simulink library "Microgrid_Library"  (R2020a) (continued)
== system Microgrid_Library == (continued)
      mask DialogControl Container4 Prompt: House 1
      mask DialogControl Container10 Prompt: Kitchen
      mask DialogControl Container22 Prompt: Bathroom
      mask DialogControl Container20 Prompt: Bedroom 1
      mask DialogControl Container16 Prompt: Bedroom 2
      mask DialogControl Container11 Prompt: Lounge
      mask Display: image('house.jpg');
  "House 5 S  " : Subsystem  mask{V=240, t=60, K_L1_W=18, K_L1_On_1=23220, K_L1_Off_1=27120, K_L1_On_2=74160, K_L1_Off_2=82020, Dishwasher_W=1200, Dishwasher_On=36420, Fridge_W=150, T_Initial=1.35, T_Low=1.2, T_High=3.1, Jug_W=1500, Jug_On_1=25620, Jug_On_2=0, Jug_On_3=0, Toaster_W=1100, Toaster_On_1=30600, Toaster_On_2=0, N_Elements=4, Oven_On_1=65040, Oven_Off_1=69900, Shower_On_a=29100, Shower_L_a=240, Shower_On_b=39000, Shower_L_b=300, Shower_On_c=0, Shower_L_c=0, K_L3_W=18, K_L3_On_1=28800, K_L3_Off_1=29400, K_L3_On_2=38700, K_L3_Off_2=39120, HW_W=2000, M=135, D=0.488, L=1.35, Ti=7, Ta=20, T_Low_WH=56, T_High_WH=58, Clock_1_W=3, Lamp_1_W=18, L1_On_1=76560, L1_Off_1=82920, L1_On_2=0, L1_Off_2=0, K_L4_W=40, K_L4_On_1=73800, K_L4_Off_1=76200, Clock_2_W=5, Lamp_2_W=18, L2_On_1=26640, L2_Off_1=29520, L2_On_2=72000, L2_Off_2=79560, K_L5_W=60, K_L5_On_1=77100, K_L5_Off_1=79560, K_L5_On_2=0, K_L5_Off_2=0, Phone_C_1_W=5, Phone_C_1_On_a=0, Phone_C_1_Off_a=8760, Phone_C_1_On_b=77100, Phone_C_1_Off_b=86400, Phone_C_2_W=6, Phone_C_2_On_a=0, Phone_C_2_Off_a=21600, Phone_C_2_On_b=77100, Phone_C_2_Off_b=86400, Phone_C_3_W=5, Phone_C_3_On_a=0, Phone_C_3_Off_a=14820, Phone_C_3_On_b=77100, Phone_C_3_Off_b=86400, Laptop_C_1_W=65, Laptop_C_1_On_a=34200, Laptop_C_1_Off_a=52200, Laptop_C_2_W=65, Laptop_C_2_On_a=72780, Laptop_C_2_Off_a=77100, Laptop_C_2_On_b=0, Laptop_C_2_Off_b=0, Lamp_3_W=24, L3_On_1=25200, L3_Off_1=60450, L3_On_2=80040, L3_Off_2=84000, K_L6_W=100, K_L6_On_1=76200, K_L6_Off_1=69800, Heater_W=500, Heater_On_1=29160, Heater_Off_1=76140, Stereo_W=50, Stereo_On_1=43560, Stereo_Off_1=45480, TV_W=200, TV_On_1=39900, TV_Off_1=52200, TV_On_2=69600, TV_Off_2=77100}
      mask prompts: V "Voltage"; t "Time"; K_L1_W "Light_1_W"; K_L1_On_1 "Light_1_On_a"; K_L1_Off_1 "Light_1_Off_a"; K_L1_On_2 "Light_1_On_b"; K_L1_Off_2 "Light_1_Off_b"; T_Initial "Fridge_Initial_Temp"; T_Low "Fridge_T_Low"; T_High "Fridge_T_High"; N_Elements "Oven_#_Elements"; Shower_L_a "Shower_Length_a"; Shower_L_b "Shower_Length_b"; Shower_L_c "Shower_Length_c"; K_L3_W "Light_3_W"; K_L3_On_1 "Light_3_On_a"; K_L3_Off_1 "Light_3_Off_a"; K_L3_On_2 "Light_3_On_b"; K_L3_Off_2 "Light_3_Off_b"; M "Mass of water"; D "HW_Diameter"; L "HW_Length"; Ti "Inlet Temp"; Ta "Ambient Temp"; L1_On_1 "Lamp_1_On_1"; L1_Off_1 "Lamp_1_Off_1"; L1_On_2 "Lamp_1_On_2"; L1_Off_2 "Lamp_1_Off_2"; K_L4_W "Light_4_W"; K_L4_On_1 "Light_4_On_a"; K_L4_Off_1 "Light_4_Off_a"; L2_On_1 "Lamp_2_On_1"; L2_Off_1 "Lamp_2_Off_1"; L2_On_2 "Lamp_2_On_2"; L2_Off_2 "Lamp_2_Off_2"; K_L5_W "Light_5_W"; K_L5_On_1 "Light_5_On_a"; K_L5_Off_1 "Light_5_Off_a"; K_L5_On_2 "Light_5_On_b"; K_L5_Off_2 "Light_5_Off_b"; L3_On_1 "Lamp_3_On_1"; L3_Off_1 "Lamp_3_Off_1"; L3_On_2 "Lamp_3_On_2"; L3_Off_2 "Lamp_3_Off_2"; K_L6_W "Light_6_W"; K_L6_On_1 "Light_6_On_a"; K_L6_Off_1 "Light_6_Off_a"
      mask DialogControl Container4 Prompt: House 1
      mask DialogControl Container10 Prompt: Kitchen
      mask DialogControl Container22 Prompt: Bathroom
      mask DialogControl Container20 Prompt: Bedroom 1
      mask DialogControl Container16 Prompt: Bedroom 2
      mask DialogControl Container11 Prompt: Lounge
      mask Display: image('house.jpg');
  House 5 W : Subsystem  mask{V=240, t=60, K_L1_W=18, K_L1_On_1=23220, K_L1_Off_1=27120, K_L1_On_2=74160, K_L1_Off_2=82020, Dishwasher_W=1200, Dishwasher_On=36420, Fridge_W=150, T_Initial=1.35, T_Low=1.2, T_High=3.1, Jug_W=1500, Jug_On_1=25620, Jug_On_2=0, Jug_On_3=0, Toaster_W=1100, Toaster_On_1=30600, Toaster_On_2=0, N_Elements=4, Oven_On_1=65040, Oven_Off_1=69900, Shower_On_a=29100, Shower_L_a=240, Shower_On_b=39000, Shower_L_b=300, Shower_On_c=0, Shower_L_c=0, K_L3_W=18, K_L3_On_1=28800, K_L3_Off_1=29400, K_L3_On_2=38700, K_L3_Off_2=39120, HW_W=2000, M=135, D=0.488, L=1.35, Ti=7, Ta=20, T_Low_WH=56, T_High_WH=58, Clock_1_W=3, Lamp_1_W=18, L1_On_1=70560, L1_Off_1=82920, L1_On_2=0, L1_Off_2=0, K_L4_W=40, K_L4_On_1=65400, K_L4_Off_1=76200, Clock_2_W=5, Lamp_2_W=18, L2_On_1=26640, L2_Off_1=29520, L2_On_2=66000, L2_Off_2=79560, K_L5_W=60, K_L5_On_1=77100, K_L5_Off_1=79560, K_L5_On_2=0, K_L5_Off_2=0, Phone_C_1_W=5, Phone_C_1_On_a=0, Phone_C_1_Off_a=8760, Phone_C_1_On_b=77100, Phone_C_1_Off_b=86400, Phone_C_2_W=6, Phone_C_2_On_a=0, Phone_C_2_Off_a=21600, Phone_C_2_On_b=77100, Phone_C_2_Off_b=86400, Phone_C_3_W=5, Phone_C_3_On_a=0, Phone_C_3_Off_a=14820, Phone_C_3_On_b=77100, Phone_C_3_Off_b=86400, Laptop_C_1_W=65, Laptop_C_1_On_a=34200, Laptop_C_1_Off_a=52200, Laptop_C_2_W=65, Laptop_C_2_On_a=72780, Laptop_C_2_Off_a=77100, Laptop_C_2_On_b=0, Laptop_C_2_Off_b=0, Lamp_3_W=24, L3_On_1=25200, L3_Off_1=27000, L3_On_2=20040, L3_Off_2=84000, K_L6_W=100, K_L6_On_1=70200, K_L6_Off_1=73800, Heater_W=2000, Heater_On_1=29160, Heater_Off_1=76140, Stereo_W=50, Stereo_On_1=43560, Stereo_Off_1=45480, TV_W=200, TV_On_1=39900, TV_Off_1=52200, TV_On_2=69600, TV_Off_2=77100}
      mask prompts: V "Voltage"; t "Time"; K_L1_W "Light_1_W"; K_L1_On_1 "Light_1_On_a"; K_L1_Off_1 "Light_1_Off_a"; K_L1_On_2 "Light_1_On_b"; K_L1_Off_2 "Light_1_Off_b"; T_Initial "Fridge_Initial_Temp"; T_Low "Fridge_T_Low"; T_High "Fridge_T_High"; N_Elements "Oven_#_Elements"; Shower_L_a "Shower_Length_a"; Shower_L_b "Shower_Length_b"; Shower_L_c "Shower_Length_c"; K_L3_W "Light_3_W"; K_L3_On_1 "Light_3_On_a"; K_L3_Off_1 "Light_3_Off_a"; K_L3_On_2 "Light_3_On_b"; K_L3_Off_2 "Light_3_Off_b"; M "Mass of water"; D "HW_Diameter"; L "HW_Length"; Ti "Inlet Temp"; Ta "Ambient Temp"; L1_On_1 "Lamp_1_On_1"; L1_Off_1 "Lamp_1_Off_1"; L1_On_2 "Lamp_1_On_2"; L1_Off_2 "Lamp_1_Off_2"; K_L4_W "Light_4_W"; K_L4_On_1 "Light_4_On_a"; K_L4_Off_1 "Light_4_Off_a"; L2_On_1 "Lamp_2_On_1"; L2_Off_1 "Lamp_2_Off_1"; L2_On_2 "Lamp_2_On_2"; L2_Off_2 "Lamp_2_Off_2"; K_L5_W "Light_5_W"; K_L5_On_1 "Light_5_On_a"; K_L5_Off_1 "Light_5_Off_a"; K_L5_On_2 "Light_5_On_b"; K_L5_Off_2 "Light_5_Off_b"; L3_On_1 "Lamp_3_On_1"; L3_Off_1 "Lamp_3_Off_1"; L3_On_2 "Lamp_3_On_2"; L3_Off_2 "Lamp_3_Off_2"; K_L6_W "Light_6_W"; K_L6_On_1 "Light_6_On_a"; K_L6_Off_1 "Light_6_Off_a"
      mask DialogControl Container4 Prompt: House 1
      mask DialogControl Container10 Prompt: Kitchen
      mask DialogControl Container22 Prompt: Bathroom
      mask DialogControl Container20 Prompt: Bedroom 1
      mask DialogControl Container16 Prompt: Bedroom 2
      mask DialogControl Container11 Prompt: Lounge
      mask Display: image('house.jpg');
  House 6 S : Subsystem  mask{V=240, t=60, K_L1_W=40, K_L1_On_1=75000, K_L1_Off_1=81300, K_L1_On_2=0, K_L1_Off_2=0, Dishwasher_W=1200, Dishwasher_On=69000, Fridge_W=360, T_Initial=1.8, T_Low=1.5, T_High=2.4, Jug_W=2000, Jug_On_1=30540, Jug_On_2=70680, Jug_On_3=0, Toaster_W=1200, Toaster_On_1=28800, Toaster_On_2=29100, N_Elements=4, Oven_On_1=61260, Oven_Off_1=65160, Shower_On_a=68760, Shower_L_a=300, Shower_On_b=77520, Shower_L_b=180, Shower_On_c=78780, Shower_L_c=300, K_L3_W=24, K_L3_On_1=68700, K_L3_Off_1=72000, K_L3_On_2=77400, K_L3_Off_2=79200, HW_W=3000, M=300, D=0.58, L=1.825, Ti=7, Ta=20, T_Low_WH=55, T_High_WH=60, Clock_1_W=6, Lamp_1_W=24, L1_On_1=21600, L1_Off_1=27000, L1_On_2=0, L1_Off_2=0, K_L4_W=0, K_L4_On_1=0, K_L4_Off_1=0, Clock_2_W=5, Lamp_2_W=24, L2_On_1=21600, L2_Off_1=27000, L2_On_2=74280, L2_Off_2=81300, K_L5_W=40, K_L5_On_1=0, K_L5_Off_1=3240, K_L5_On_2=78600, K_L5_Off_2=86400, Phone_C_1_W=6, Phone_C_1_On_a=57600, Phone_C_1_Off_a=81600, Phone_C_1_On_b=0, Phone_C_1_Off_b=0, Phone_C_2_W=5, Phone_C_2_On_a=0, Phone_C_2_Off_a=12000, Phone_C_2_On_b=84000, Phone_C_2_Off_b=86400, Phone_C_3_W=0, Phone_C_3_On_a=0, Phone_C_3_Off_a=0, Phone_C_3_On_b=0, Phone_C_3_Off_b=0, Laptop_C_1_W=65, Laptop_C_1_On_a=75600, Laptop_C_1_Off_a=79560, Laptop_C_2_W=55, Laptop_C_2_On_a=0, Laptop_C_2_Off_a=3120, Laptop_C_2_On_b=79440, Laptop_C_2_Off_b=86400, Lamp_3_W=40, L3_On_1=21720, L3_Off_1=30000, L3_On_2=72000, L3_Off_2=81000, K_L6_W=60, K_L6_On_1=72000, K_L6_Off_1=81000, Heater_W=2100, Heater_On_1=56340, Heater_Off_1=73020, Stereo_W=100, Stereo_On_1=63000, Stereo_Off_1=64800, TV_W=400, TV_On_1=22020, TV_Off_1=70200, TV_On_2=70200, TV_Off_2=78600}
      mask prompts: V "Voltage"; t "Time"; K_L1_W "Light_1_W"; K_L1_On_1 "Light_1_On_a"; K_L1_Off_1 "Light_1_Off_a"; K_L1_On_2 "Light_1_On_b"; K_L1_Off_2 "Light_1_Off_b"; T_Initial "Fridge_Initial_Temp"; T_Low "Fridge_T_Low"; T_High "Fridge_T_High"; N_Elements "Oven_#_Elements"; Shower_L_a "Shower_Length_a"; Shower_L_b "Shower_Length_b"; Shower_L_c "Shower_Length_c"; K_L3_W "Light_3_W"; K_L3_On_1 "Light_3_On_a"; K_L3_Off_1 "Light_3_Off_a"; K_L3_On_2 "Light_3_On_b"; K_L3_Off_2 "Light_3_Off_b"; M "Mass of water"; D "HW_Diameter"; L "HW_Length"; Ti "Inlet Temp"; Ta "Ambient Temp"; L1_On_1 "Lamp_1_On_1"; L1_Off_1 "Lamp_1_Off_1"; L1_On_2 "Lamp_1_On_2"; L1_Off_2 "Lamp_1_Off_2"; K_L4_W "Light_4_W"; K_L4_On_1 "Light_4_On_a"; K_L4_Off_1 "Light_4_Off_a"; L2_On_1 "Lamp_2_On_1"; L2_Off_1 "Lamp_2_Off_1"; L2_On_2 "Lamp_2_On_2"; L2_Off_2 "Lamp_2_Off_2"; K_L5_W "Light_5_W"; K_L5_On_1 "Light_5_On_a"; K_L5_Off_1 "Light_5_Off_a"; K_L5_On_2 "Light_5_On_b"; K_L5_Off_2 "Light_5_Off_b"; L3_On_1 "Lamp_3_On_1"; L3_Off_1 "Lamp_3_Off_1"; L3_On_2 "Lamp_3_On_2"; L3_Off_2 "Lamp_3_Off_2"; K_L6_W "Light_6_W"; K_L6_On_1 "Light_6_On_a"; K_L6_Off_1 "Light_6_Off_a"
      mask DialogControl Container4 Prompt: House 1
      mask DialogControl Container10 Prompt: Kitchen
      mask DialogControl Container22 Prompt: Bathroom
      mask DialogControl Container20 Prompt: Bedroom 1
      mask DialogControl Container16 Prompt: Bedroom 2
      mask DialogControl Container11 Prompt: Lounge
      mask Display: image('house.jpg');
  House 6 W : Subsystem  mask{V=240, t=60, K_L1_W=40, K_L1_On_1=63000, K_L1_Off_1=69300, K_L1_On_2=0, K_L1_Off_2=0, Dishwasher_W=1200, Dishwasher_On=69000, Fridge_W=360, T_Initial=1.8, T_Low=1.5, T_High=2.4, Jug_W=2000, Jug_On_1=30540, Jug_On_2=70680, Jug_On_3=0, Toaster_W=1200, Toaster_On_1=28800, Toaster_On_2=29100, N_Elements=4, Oven_On_1=61260, Oven_Off_1=65160, Shower_On_a=68760, Shower_L_a=300, Shower_On_b=77520, Shower_L_b=180, Shower_On_c=78780, Shower_L_c=300, K_L3_W=24, K_L3_On_1=68700, K_L3_Off_1=72000, K_L3_On_2=77400, K_L3_Off_2=79200, HW_W=3000, M=300, D=0.58, L=1.825, Ti=7, Ta=20, T_Low_WH=55, T_High_WH=60, Clock_1_W=6, Lamp_1_W=24, L1_On_1=21600, L1_Off_1=27000, L1_On_2=0, L1_Off_2=0, K_L4_W=40, K_L4_On_1=1150, K_L4_Off_1=1200, Clock_2_W=5, Lamp_2_W=24, L2_On_1=21600, L2_Off_1=27000, L2_On_2=74280, L2_Off_2=81300, K_L5_W=40, K_L5_On_1=0, K_L5_Off_1=3240, K_L5_On_2=78600, K_L5_Off_2=86400, Phone_C_1_W=6, Phone_C_1_On_a=57600, Phone_C_1_Off_a=81600, Phone_C_1_On_b=0, Phone_C_1_Off_b=0, Phone_C_2_W=5, Phone_C_2_On_a=0, Phone_C_2_Off_a=12000, Phone_C_2_On_b=84000, Phone_C_2_Off_b=86400, Phone_C_3_W=0, Phone_C_3_On_a=0, Phone_C_3_Off_a=0, Phone_C_3_On_b=0, Phone_C_3_Off_b=0, Laptop_C_1_W=65, Laptop_C_1_On_a=75600, Laptop_C_1_Off_a=79560, Laptop_C_2_W=55, Laptop_C_2_On_a=0, Laptop_C_2_Off_a=3120, Laptop_C_2_On_b=79440, Laptop_C_2_Off_b=86400, Lamp_3_W=40, L3_On_1=21720, L3_Off_1=30000, L3_On_2=66000, L3_Off_2=81000, K_L6_W=60, K_L6_On_1=66000, K_L6_Off_1=81000, Heater_W=2100, Heater_On_1=56340, Heater_Off_1=73020, Stereo_W=100, Stereo_On_1=63000, Stereo_Off_1=64800, TV_W=400, TV_On_1=22020, TV_Off_1=70200, TV_On_2=70200, TV_Off_2=78600}
      mask prompts: V "Voltage"; t "Time"; K_L1_W "Light_1_W"; K_L1_On_1 "Light_1_On_a"; K_L1_Off_1 "Light_1_Off_a"; K_L1_On_2 "Light_1_On_b"; K_L1_Off_2 "Light_1_Off_b"; T_Initial "Fridge_Initial_Temp"; T_Low "Fridge_T_Low"; T_High "Fridge_T_High"; N_Elements "Oven_#_Elements"; Shower_L_a "Shower_Length_a"; Shower_L_b "Shower_Length_b"; Shower_L_c "Shower_Length_c"; K_L3_W "Light_3_W"; K_L3_On_1 "Light_3_On_a"; K_L3_Off_1 "Light_3_Off_a"; K_L3_On_2 "Light_3_On_b"; K_L3_Off_2 "Light_3_Off_b"; M "Mass of water"; D "HW_Diameter"; L "HW_Length"; Ti "Inlet Temp"; Ta "Ambient Temp"; L1_On_1 "Lamp_1_On_1"; L1_Off_1 "Lamp_1_Off_1"; L1_On_2 "Lamp_1_On_2"; L1_Off_2 "Lamp_1_Off_2"; K_L4_W "Light_4_W"; K_L4_On_1 "Light_4_On_a"; K_L4_Off_1 "Light_4_Off_a"; L2_On_1 "Lamp_2_On_1"; L2_Off_1 "Lamp_2_Off_1"; L2_On_2 "Lamp_2_On_2"; L2_Off_2 "Lamp_2_Off_2"; K_L5_W "Light_5_W"; K_L5_On_1 "Light_5_On_a"; K_L5_Off_1 "Light_5_Off_a"; K_L5_On_2 "Light_5_On_b"; K_L5_Off_2 "Light_5_Off_b"; L3_On_1 "Lamp_3_On_1"; L3_Off_1 "Lamp_3_Off_1"; L3_On_2 "Lamp_3_On_2"; L3_Off_2 "Lamp_3_Off_2"; K_L6_W "Light_6_W"; K_L6_On_1 "Light_6_On_a"; K_L6_Off_1 "Light_6_Off_a"
      mask DialogControl Container4 Prompt: House 1
      mask DialogControl Container10 Prompt: Kitchen
      mask DialogControl Container22 Prompt: Bathroom
      mask DialogControl Container20 Prompt: Bedroom 1
      mask DialogControl Container16 Prompt: Bedroom 2
      mask DialogControl Container11 Prompt: Lounge
      mask Display: image('house.jpg');
  House 7 S : Subsystem  mask{V=240, t=60, K_L1_W=60, K_L1_On_1=27120, K_L1_Off_1=27780, K_L1_On_2=73140, K_L1_Off_2=78000, Dishwasher_W=1200, Dishwasher_On=28800, Fridge_W=200, T_Initial=1, T_Low=1.3, T_High=2, Jug_W=1500, Jug_On_1=28980, Jug_On_2=48060, Jug_On_3=0, Toaster_W=1100, Toaster_On_1=64800, Toaster_On_2=0, N_Elements=3, Oven_On_1=68640, Oven_Off_1=70860, Shower_On_a=30720, Shower_L_a=300, Shower_On_b=75450, Shower_L_b=240, Shower_On_c=0, Shower_L_c=0, K_L3_W=40, K_L3_On_1=72120, K_L3_Off_1=72600, K_L3_On_2=0, K_L3_Off_2=0, HW_W=2000, M=180, D=0.58, L=1.166, Ti=7, Ta=20, T_Low_WH=57, T_High_WH=62, Clock_1_W=4, Lamp_1_W=24, L1_On_1=72540, L1_Off_1=72900, L1_On_2=72540, L1_Off_2=72900, K_L4_W=0, K_L4_On_1=0, K_L4_Off_1=0, Clock_2_W=4, Lamp_2_W=40, L2_On_1=27000, L2_Off_1=31560, L2_On_2=77160, L2_Off_2=85200, K_L5_W=80, K_L5_On_1=27000, K_L5_Off_1=28200, K_L5_On_2=83160, K_L5_Off_2=83940, Phone_C_1_W=4, Phone_C_1_On_a=0, Phone_C_1_Off_a=28380, Phone_C_1_On_b=78900, Phone_C_1_Off_b=86400, Phone_C_2_W=6, Phone_C_2_On_a=30240, Phone_C_2_Off_a=51840, Phone_C_2_On_b=0, Phone_C_2_Off_b=0, Phone_C_3_W=4, Phone_C_3_On_a=0, Phone_C_3_Off_a=24360, Phone_C_3_On_b=85200, Phone_C_3_Off_b=86400, Laptop_C_1_W=55, Laptop_C_1_On_a=36240, Laptop_C_1_Off_a=50400, Laptop_C_2_W=45, Laptop_C_2_On_a=0, Laptop_C_2_Off_a=22860, Laptop_C_2_On_b=84600, Laptop_C_2_Off_b=86400, Lamp_3_W=0, L3_On_1=0, L3_Off_1=0, L3_On_2=0, L3_Off_2=0, K_L6_W=18, K_L6_On_1=71940, K_L6_Off_1=79200, Heater_W=1000, Heater_On_1=54000, Heater_Off_1=73800, Stereo_W=50, Stereo_On_1=36540, Stereo_Off_1=50100, TV_W=100, TV_On_1=55260, TV_Off_1=57780, TV_On_2=72000, TV_Off_2=75840}
      mask prompts: V "Voltage"; t "Time"; K_L1_W "Light_1_W"; K_L1_On_1 "Light_1_On_a"; K_L1_Off_1 "Light_1_Off_a"; K_L1_On_2 "Light_1_On_b"; K_L1_Off_2 "Light_1_Off_b"; T_Initial "Fridge_Initial_Temp"; T_Low "Fridge_T_Low"; T_High "Fridge_T_High"; N_Elements "Oven_#_Elements"; Shower_L_a "Shower_Length_a"; Shower_L_b "Shower_Length_b"; Shower_L_c "Shower_Length_c"; K_L3_W "Light_3_W"; K_L3_On_1 "Light_3_On_a"; K_L3_Off_1 "Light_3_Off_a"; K_L3_On_2 "Light_3_On_b"; K_L3_Off_2 "Light_3_Off_b"; M "Mass of water"; D "HW_Diameter"; L "HW_Length"; Ti "Inlet Temp"; Ta "Ambient Temp"; L1_On_1 "Lamp_1_On_1"; L1_Off_1 "Lamp_1_Off_1"; L1_On_2 "Lamp_1_On_2"; L1_Off_2 "Lamp_1_Off_2"; K_L4_W "Light_4_W"; K_L4_On_1 "Light_4_On_a"; K_L4_Off_1 "Light_4_Off_a"; L2_On_1 "Lamp_2_On_1"; L2_Off_1 "Lamp_2_Off_1"; L2_On_2 "Lamp_2_On_2"; L2_Off_2 "Lamp_2_Off_2"; K_L5_W "Light_5_W"; K_L5_On_1 "Light_5_On_a"; K_L5_Off_1 "Light_5_Off_a"; K_L5_On_2 "Light_5_On_b"; K_L5_Off_2 "Light_5_Off_b"; L3_On_1 "Lamp_3_On_1"; L3_Off_1 "Lamp_3_Off_1"; L3_On_2 "Lamp_3_On_2"; L3_Off_2 "Lamp_3_Off_2"; K_L6_W "Light_6_W"; K_L6_On_1 "Light_6_On_a"; K_L6_Off_1 "Light_6_Off_a"
      mask DialogControl Container4 Prompt: House 1
      mask DialogControl Container10 Prompt: Kitchen
      mask DialogControl Container22 Prompt: Bathroom
      mask DialogControl Container20 Prompt: Bedroom 1
      mask DialogControl Container16 Prompt: Bedroom 2
      mask DialogControl Container11 Prompt: Lounge
      mask Display: image('house.jpg');
  House 7 W : Subsystem  mask{V=240, t=60, K_L1_W=60, K_L1_On_1=27120, K_L1_Off_1=27780, K_L1_On_2=67140, K_L1_Off_2=72000, Dishwasher_W=1200, Dishwasher_On=28800, Fridge_W=200, T_Initial=1, T_Low=1.3, T_High=2, Jug_W=1500, Jug_On_1=28980, Jug_On_2=48060, Jug_On_3=0, Toaster_W=1100, Toaster_On_1=64800, Toaster_On_2=0, N_Elements=3, Oven_On_1=68640, Oven_Off_1=70860, Shower_On_a=30720, Shower_L_a=300, Shower_On_b=75450, Shower_L_b=240, Shower_On_c=0, Shower_L_c=0, K_L3_W=40, K_L3_On_1=72120, K_L3_Off_1=72600, K_L3_On_2=0, K_L3_Off_2=0, HW_W=2000, M=180, D=0.58, L=1.166, Ti=7, Ta=20, T_Low_WH=57, T_High_WH=62, Clock_1_W=4, Lamp_1_W=24, L1_On_1=72540, L1_Off_1=72900, L1_On_2=72540, L1_Off_2=72900, K_L4_W=0, K_L4_On_1=0, K_L4_Off_1=0, Clock_2_W=4, Lamp_2_W=40, L2_On_1=27000, L2_Off_1=31560, L2_On_2=77160, L2_Off_2=85200, K_L5_W=80, K_L5_On_1=27000, K_L5_Off_1=28200, K_L5_On_2=83160, K_L5_Off_2=83940, Phone_C_1_W=4, Phone_C_1_On_a=0, Phone_C_1_Off_a=28380, Phone_C_1_On_b=78900, Phone_C_1_Off_b=86400, Phone_C_2_W=6, Phone_C_2_On_a=30240, Phone_C_2_Off_a=51840, Phone_C_2_On_b=0, Phone_C_2_Off_b=0, Phone_C_3_W=4, Phone_C_3_On_a=0, Phone_C_3_Off_a=24360, Phone_C_3_On_b=85200, Phone_C_3_Off_b=86400, Laptop_C_1_W=55, Laptop_C_1_On_a=36240, Laptop_C_1_Off_a=50400, Laptop_C_2_W=45, Laptop_C_2_On_a=0, Laptop_C_2_Off_a=22860, Laptop_C_2_On_b=84600, Laptop_C_2_Off_b=86400, Lamp_3_W=0, L3_On_1=0, L3_Off_1=0, L3_On_2=0, L3_Off_2=0, K_L6_W=18, K_L6_On_1=71940, K_L6_Off_1=79200, Heater_W=1000, Heater_On_1=54000, Heater_Off_1=73800, Stereo_W=50, Stereo_On_1=36540, Stereo_Off_1=50100, TV_W=100, TV_On_1=55260, TV_Off_1=57780, TV_On_2=72000, TV_Off_2=75840}
      mask prompts: V "Voltage"; t "Time"; K_L1_W "Light_1_W"; K_L1_On_1 "Light_1_On_a"; K_L1_Off_1 "Light_1_Off_a"; K_L1_On_2 "Light_1_On_b"; K_L1_Off_2 "Light_1_Off_b"; T_Initial "Fridge_Initial_Temp"; T_Low "Fridge_T_Low"; T_High "Fridge_T_High"; N_Elements "Oven_#_Elements"; Shower_L_a "Shower_Length_a"; Shower_L_b "Shower_Length_b"; Shower_L_c "Shower_Length_c"; K_L3_W "Light_3_W"; K_L3_On_1 "Light_3_On_a"; K_L3_Off_1 "Light_3_Off_a"; K_L3_On_2 "Light_3_On_b"; K_L3_Off_2 "Light_3_Off_b"; M "Mass of water"; D "HW_Diameter"; L "HW_Length"; Ti "Inlet Temp"; Ta "Ambient Temp"; L1_On_1 "Lamp_1_On_1"; L1_Off_1 "Lamp_1_Off_1"; L1_On_2 "Lamp_1_On_2"; L1_Off_2 "Lamp_1_Off_2"; K_L4_W "Light_4_W"; K_L4_On_1 "Light_4_On_a"; K_L4_Off_1 "Light_4_Off_a"; L2_On_1 "Lamp_2_On_1"; L2_Off_1 "Lamp_2_Off_1"; L2_On_2 "Lamp_2_On_2"; L2_Off_2 "Lamp_2_Off_2"; K_L5_W "Light_5_W"; K_L5_On_1 "Light_5_On_a"; K_L5_Off_1 "Light_5_Off_a"; K_L5_On_2 "Light_5_On_b"; K_L5_Off_2 "Light_5_Off_b"; L3_On_1 "Lamp_3_On_1"; L3_Off_1 "Lamp_3_Off_1"; L3_On_2 "Lamp_3_On_2"; L3_Off_2 "Lamp_3_Off_2"; K_L6_W "Light_6_W"; K_L6_On_1 "Light_6_On_a"; K_L6_Off_1 "Light_6_Off_a"
      mask DialogControl Container4 Prompt: House 1
      mask DialogControl Container10 Prompt: Kitchen
      mask DialogControl Container22 Prompt: Bathroom
      mask DialogControl Container20 Prompt: Bedroom 1
      mask DialogControl Container16 Prompt: Bedroom 2
      mask DialogControl Container11 Prompt: Lounge
      mask Display: image('house.jpg');
  House 8 S : Subsystem  mask{V=240, t=60, K_L1_W=24, K_L1_On_1=75480, K_L1_Off_1=81540, K_L1_On_2=0, K_L1_Off_2=0, K_L2_W=60, K_L2_On_1=27540, K_L2_Off_1=31440, Fridge_W=200, T_Initial=1.7, T_Low=0.9, T_High=3.6, Jug_W=2000, Jug_On_1=32580, Jug_On_2=0, Jug_On_3=0, Toaster_W=1100, Toaster_On_1=33120, Toaster_On_2=0, N_Elements=4, Oven_On_1=64420, Oven_Off_1=67440, Shower_On_a=31200, Shower_L_a=240, Shower_On_b=68700, Shower_L_b=300, Shower_On_c=83340, Shower_L_c=180, K_L3_W=100, K_L3_On_1=68400, K_L3_Off_1=69000, K_L3_On_2=83280, K_L3_Off_2=83700, HW_W=3000, M=250, D=0.58, L=1.56, Ti=7, Ta=20, T_Low_WH=55.1, T_High_WH=64, Clock_1_W=5, Lamp_1_W=24, L1_On_1=74400, L1_Off_1=75000, L1_On_2=0, L1_Off_2=0, K_L4_W=40, K_L4_On_1=21900, K_L4_Off_1=27240, Clock_2_W=6, Lamp_2_W=60, L2_On_1=0, L2_Off_1=1380, L2_On_2=84180, L2_Off_2=86400, K_L5_W=24, K_L5_On_1=22200, K_L5_Off_1=24300, K_L5_On_2=83160, K_L5_Off_2=84180, Phone_C_1_W=7, Phone_C_1_On_a=0, Phone_C_1_Off_a=31920, Phone_C_1_On_b=76440, Phone_C_1_Off_b=86400, Phone_C_2_W=4, Phone_C_2_On_a=0, Phone_C_2_Off_a=18600, Phone_C_2_On_b=79140, Phone_C_2_Off_b=86400, Phone_C_3_W=0, Phone_C_3_On_a=0, Phone_C_3_Off_a=0, Phone_C_3_On_b=0, Phone_C_3_Off_b=0, Laptop_C_1_W=65, Laptop_C_1_On_a=22800, Laptop_C_1_Off_a=85020, Laptop_C_2_W=45, Laptop_C_2_On_a=0, Laptop_C_2_Off_a=27360, Laptop_C_2_On_b=78720, Laptop_C_2_Off_b=86400, Lamp_3_W=40, L3_On_1=22080, L3_Off_1=27000, L3_On_2=72480, L3_Off_2=78360, K_L6_W=24, K_L6_On_1=78360, K_L6_Off_1=8400, Heater_W=2000, Heater_On_1=58320, Heater_Off_1=72360, Stereo_W=80, Stereo_On_1=57600, Stereo_Off_1=64800, TV_W=200, TV_On_1=23820, TV_Off_1=27000, TV_On_2=73980, TV_Off_2=83160}
      mask prompts: V "Voltage"; t "Time"; K_L1_W "Light_1_W"; K_L1_On_1 "Light_1_On_a"; K_L1_Off_1 "Light_1_Off_a"; K_L1_On_2 "Light_1_On_b"; K_L1_Off_2 "Light_1_Off_b"; K_L2_W "Light_2_W"; K_L2_On_1 "Light_2_On_a"; K_L2_Off_1 "Light_2_Off_a"; T_Initial "Fridge_Initial_Temp"; T_Low "Fridge_T_Low"; T_High "Fridge_T_High"; N_Elements "Oven_#_Elements"; Shower_L_a "Shower_Length_a"; Shower_L_b "Shower_Length_b"; Shower_L_c "Shower_Length_c"; K_L3_W "Light_3_W"; K_L3_On_1 "Light_3_On_a"; K_L3_Off_1 "Light_3_Off_a"; K_L3_On_2 "Light_3_On_b"; K_L3_Off_2 "Light_3_Off_b"; M "Mass of water"; D "HW_Diameter"; L "HW_Length"; Ti "Inlet Temp"; Ta "Ambient Temp"; L1_On_1 "Lamp_1_On_1"; L1_Off_1 "Lamp_1_Off_1"; L1_On_2 "Lamp_1_On_2"; L1_Off_2 "Lamp_1_Off_2"; K_L4_W "Light_4_W"; K_L4_On_1 "Light_4_On_a"; K_L4_Off_1 "Light_4_Off_a"; L2_On_1 "Lamp_2_On_1"; L2_Off_1 "Lamp_2_Off_1"; L2_On_2 "Lamp_2_On_2"; L2_Off_2 "Lamp_2_Off_2"; K_L5_W "Light_5_W"; K_L5_On_1 "Light_5_On_a"; K_L5_Off_1 "Light_5_Off_a"; K_L5_On_2 "Light_5_On_b"; K_L5_Off_2 "Light_5_Off_b"; L3_On_1 "Lamp_3_On_1"; L3_Off_1 "Lamp_3_Off_1"; L3_On_2 "Lamp_3_On_2"; L3_Off_2 "Lamp_3_Off_2"; K_L6_W "Light_6_W"; K_L6_On_1 "Light_6_On_a"; K_L6_Off_1 "Light_6_Off_a"
      mask DialogControl Container4 Prompt: House 1
      mask DialogControl Container10 Prompt: Kitchen
      mask DialogControl Container22 Prompt: Bathroom
      mask DialogControl Container20 Prompt: Bedroom 1
      mask DialogControl Container16 Prompt: Bedroom 2
      mask DialogControl Container11 Prompt: Lounge
      mask Display: image('house.jpg');
  House 8 W : Subsystem  mask{V=240, t=60, K_L1_W=24, K_L1_On_1=63480, K_L1_Off_1=69540, K_L1_On_2=0, K_L1_Off_2=0, K_L2_W=60, K_L2_On_1=27540, K_L2_Off_1=31440, Fridge_W=200, T_Initial=1.7, T_Low=0.9, T_High=3.6, Jug_W=2000, Jug_On_1=32580, Jug_On_2=0, Jug_On_3=0, Toaster_W=1100, Toaster_On_1=33120, Toaster_On_2=0, N_Elements=4, Oven_On_1=64420, Oven_Off_1=67440, Shower_On_a=31200, Shower_L_a=240, Shower_On_b=68700, Shower_L_b=300, Shower_On_c=83340, Shower_L_c=180, K_L3_W=100, K_L3_On_1=68400, K_L3_Off_1=69000, K_L3_On_2=83280, K_L3_Off_2=83700, HW_W=3000, M=250, D=0.58, L=1.56, Ti=7, Ta=20, T_Low_WH=55.1, T_High_WH=64, Clock_1_W=5, Lamp_1_W=24, L1_On_1=68400, L1_Off_1=69000, L1_On_2=0, L1_Off_2=0, K_L4_W=40, K_L4_On_1=21900, K_L4_Off_1=27240, Clock_2_W=6, Lamp_2_W=60, L2_On_1=0, L2_Off_1=1380, L2_On_2=84180, L2_Off_2=86400, K_L5_W=24, K_L5_On_1=22200, K_L5_Off_1=24300, K_L5_On_2=83160, K_L5_Off_2=84180, Phone_C_1_W=7, Phone_C_1_On_a=0, Phone_C_1_Off_a=31920, Phone_C_1_On_b=76440, Phone_C_1_Off_b=86400, Phone_C_2_W=4, Phone_C_2_On_a=0, Phone_C_2_Off_a=18600, Phone_C_2_On_b=79140, Phone_C_2_Off_b=86400, Phone_C_3_W=0, Phone_C_3_On_a=0, Phone_C_3_Off_a=0, Phone_C_3_On_b=0, Phone_C_3_Off_b=0, Laptop_C_1_W=65, Laptop_C_1_On_a=22800, Laptop_C_1_Off_a=85020, Laptop_C_2_W=45, Laptop_C_2_On_a=0, Laptop_C_2_Off_a=27360, Laptop_C_2_On_b=78720, Laptop_C_2_Off_b=86400, Lamp_3_W=40, L3_On_1=22080, L3_Off_1=27000, L3_On_2=72480, L3_Off_2=78360, K_L6_W=24, K_L6_On_1=78360, K_L6_Off_1=8400, Heater_W=2000, Heater_On_1=58320, Heater_Off_1=72360, Stereo_W=80, Stereo_On_1=57600, Stereo_Off_1=64800, TV_W=200, TV_On_1=23820, TV_Off_1=27000, TV_On_2=73980, TV_Off_2=83160}
      mask prompts: V "Voltage"; t "Time"; K_L1_W "Light_1_W"; K_L1_On_1 "Light_1_On_a"; K_L1_Off_1 "Light_1_Off_a"; K_L1_On_2 "Light_1_On_b"; K_L1_Off_2 "Light_1_Off_b"; K_L2_W "Light_2_W"; K_L2_On_1 "Light_2_On_a"; K_L2_Off_1 "Light_2_Off_a"; T_Initial "Fridge_Initial_Temp"; T_Low "Fridge_T_Low"; T_High "Fridge_T_High"; N_Elements "Oven_#_Elements"; Shower_L_a "Shower_Length_a"; Shower_L_b "Shower_Length_b"; Shower_L_c "Shower_Length_c"; K_L3_W "Light_3_W"; K_L3_On_1 "Light_3_On_a"; K_L3_Off_1 "Light_3_Off_a"; K_L3_On_2 "Light_3_On_b"; K_L3_Off_2 "Light_3_Off_b"; M "Mass of water"; D "HW_Diameter"; L "HW_Length"; Ti "Inlet Temp"; Ta "Ambient Temp"; L1_On_1 "Lamp_1_On_1"; L1_Off_1 "Lamp_1_Off_1"; L1_On_2 "Lamp_1_On_2"; L1_Off_2 "Lamp_1_Off_2"; K_L4_W "Light_4_W"; K_L4_On_1 "Light_4_On_a"; K_L4_Off_1 "Light_4_Off_a"; L2_On_1 "Lamp_2_On_1"; L2_Off_1 "Lamp_2_Off_1"; L2_On_2 "Lamp_2_On_2"; L2_Off_2 "Lamp_2_Off_2"; K_L5_W "Light_5_W"; K_L5_On_1 "Light_5_On_a"; K_L5_Off_1 "Light_5_Off_a"; K_L5_On_2 "Light_5_On_b"; K_L5_Off_2 "Light_5_Off_b"; L3_On_1 "Lamp_3_On_1"; L3_Off_1 "Lamp_3_Off_1"; L3_On_2 "Lamp_3_On_2"; L3_Off_2 "Lamp_3_Off_2"; K_L6_W "Light_6_W"; K_L6_On_1 "Light_6_On_a"; K_L6_Off_1 "Light_6_Off_a"
      mask DialogControl Container4 Prompt: House 1
      mask DialogControl Container10 Prompt: Kitchen
      mask DialogControl Container22 Prompt: Bathroom
      mask DialogControl Container20 Prompt: Bedroom 1
      mask DialogControl Container16 Prompt: Bedroom 2
      mask DialogControl Container11 Prompt: Lounge
      mask Display: image('house.jpg');
  House 9 S : Subsystem  mask{V=240, t=60, K_L1_W=18, K_L1_On_1=21720, K_L1_Off_1=24060, K_L1_On_2=72000, K_L1_Off_2=78540, Dishwasher_W=1000, Dishwasher_On=78480, Fridge_W=360, T_Initial=1, T_Low=1.67, T_High=2.33, Jug_W=1000, Jug_On_1=21840, Jug_On_2=23520, Jug_On_3=0, Toaster_W=0, Toaster_On_1=0, Toaster_On_2=0, N_Elements=3, Oven_On_1=74040, Oven_Off_1=75540, Shower_On_a=26280, Shower_L_a=300, Shower_On_b=76620, Shower_L_b=240, Shower_On_c=78120, Shower_L_c=300, K_L3_W=60, K_L3_On_1=76500, K_L3_Off_1=76800, K_L3_On_2=77940, K_L3_Off_2=78600, HW_W=2000, M=177, D=0.488, L=1.72, Ti=7, Ta=20, T_Low_WH=55, T_High_WH=57.5, Clock_1_W=3, Lamp_1_W=18, L1_On_1=25200, L1_Off_1=29220, L1_On_2=0, L1_Off_2=0, K_L4_W=40, K_L4_On_1=76680, K_L4_Off_1=81300, Clock_2_W=6, Lamp_2_W=24, L2_On_1=24900, L2_Off_1=29400, L2_On_2=75240, L2_Off_2=77100, K_L5_W=40, K_L5_On_1=25020, K_L5_Off_1=25920, K_L5_On_2=75240, K_L5_Off_2=76080, Phone_C_1_W=6, Phone_C_1_On_a=0, Phone_C_1_Off_a=8100, Phone_C_1_On_b=79200, Phone_C_1_Off_b=86400, Phone_C_2_W=4, Phone_C_2_On_a=0, Phone_C_2_Off_a=26040, Phone_C_2_On_b=77100, Phone_C_2_Off_b=86400, Phone_C_3_W=6, Phone_C_3_On_a=0, Phone_C_3_Off_a=14100, Phone_C_3_On_b=70200, Phone_C_3_Off_b=86400, Laptop_C_1_W=35, Laptop_C_1_On_a=64560, Laptop_C_1_Off_a=71100, Laptop_C_2_W=65, Laptop_C_2_On_a=0, Laptop_C_2_Off_a=23400, Laptop_C_2_On_b=79140, Laptop_C_2_Off_b=86400, Lamp_3_W=0, L3_On_1=0, L3_Off_1=0, L3_On_2=0, L3_Off_2=0, K_L6_W=40, K_L6_On_1=73800, K_L6_Off_1=76680, Heater_W=2000, Heater_On_1=60000, Heater_Off_1=70200, Stereo_W=40, Stereo_On_1=70320, Stereo_Off_1=77520, TV_W=300, TV_On_1=64440, TV_Off_1=70200, TV_On_2=0, TV_Off_2=0}
      mask prompts: V "Voltage"; t "Time"; K_L1_W "Light_1_W"; K_L1_On_1 "Light_1_On_a"; K_L1_Off_1 "Light_1_Off_a"; K_L1_On_2 "Light_1_On_b"; K_L1_Off_2 "Light_1_Off_b"; T_Initial "Fridge_Initial_Temp"; T_Low "Fridge_T_Low"; T_High "Fridge_T_High"; N_Elements "Oven_#_Elements"; Shower_L_a "Shower_Length_a"; Shower_L_b "Shower_Length_b"; Shower_L_c "Shower_Length_c"; K_L3_W "Light_3_W"; K_L3_On_1 "Light_3_On_a"; K_L3_Off_1 "Light_3_Off_a"; K_L3_On_2 "Light_3_On_b"; K_L3_Off_2 "Light_3_Off_b"; M "Mass of water"; D "HW_Diameter"; L "HW_Length"; Ti "Inlet Temp"; Ta "Ambient Temp"; L1_On_1 "Lamp_1_On_1"; L1_Off_1 "Lamp_1_Off_1"; L1_On_2 "Lamp_1_On_2"; L1_Off_2 "Lamp_1_Off_2"; K_L4_W "Light_4_W"; K_L4_On_1 "Light_4_On_a"; K_L4_Off_1 "Light_4_Off_a"; L2_On_1 "Lamp_2_On_1"; L2_Off_1 "Lamp_2_Off_1"; L2_On_2 "Lamp_2_On_2"; L2_Off_2 "Lamp_2_Off_2"; K_L5_W "Light_5_W"; K_L5_On_1 "Light_5_On_a"; K_L5_Off_1 "Light_5_Off_a"; K_L5_On_2 "Light_5_On_b"; K_L5_Off_2 "Light_5_Off_b"; L3_On_1 "Lamp_3_On_1"; L3_Off_1 "Lamp_3_Off_1"; L3_On_2 "Lamp_3_On_2"; L3_Off_2 "Lamp_3_Off_2"; K_L6_W "Light_6_W"; K_L6_On_1 "Light_6_On_a"; K_L6_Off_1 "Light_6_Off_a"
      mask DialogControl Container4 Prompt: House 1
      mask DialogControl Container10 Prompt: Kitchen
      mask DialogControl Container22 Prompt: Bathroom
      mask DialogControl Container20 Prompt: Bedroom 1
      mask DialogControl Container16 Prompt: Bedroom 2
      mask DialogControl Container11 Prompt: Lounge
      mask Display: image('house.jpg');
  House 9 W : Subsystem  mask{V=240, t=60, K_L1_W=18, K_L1_On_1=21720, K_L1_Off_1=24060, K_L1_On_2=72000, K_L1_Off_2=78540, Dishwasher_W=1000, Dishwasher_On=78480, Fridge_W=360, T_Initial=1, T_Low=1.67, T_High=2.33, Jug_W=1000, Jug_On_1=21840, Jug_On_2=23520, Jug_On_3=0, Toaster_W=0, Toaster_On_1=0, Toaster_On_2=0, N_Elements=3, Oven_On_1=74040, Oven_Off_1=75540, Shower_On_a=26280, Shower_L_a=300, Shower_On_b=76620, Shower_L_b=240, Shower_On_c=78120, Shower_L_c=300, K_L3_W=60, K_L3_On_1=76500, K_L3_Off_1=76800, K_L3_On_2=77940, K_L3_Off_2=78600, HW_W=2000, M=177, D=0.488, L=1.72, Ti=7, Ta=20, T_Low_WH=55, T_High_WH=57.5, Clock_1_W=3, Lamp_1_W=18, L1_On_1=25200, L1_Off_1=29220, L1_On_2=0, L1_Off_2=0, K_L4_W=40, K_L4_On_1=76680, K_L4_Off_1=81300, Clock_2_W=6, Lamp_2_W=24, L2_On_1=24900, L2_Off_1=29400, L2_On_2=69240, L2_Off_2=71100, K_L5_W=40, K_L5_On_1=25020, K_L5_Off_1=25920, K_L5_On_2=69240, K_L5_Off_2=76080, Phone_C_1_W=6, Phone_C_1_On_a=0, Phone_C_1_Off_a=8100, Phone_C_1_On_b=79200, Phone_C_1_Off_b=86400, Phone_C_2_W=4, Phone_C_2_On_a=0, Phone_C_2_Off_a=26040, Phone_C_2_On_b=77100, Phone_C_2_Off_b=86400, Phone_C_3_W=6, Phone_C_3_On_a=0, Phone_C_3_Off_a=14100, Phone_C_3_On_b=70200, Phone_C_3_Off_b=86400, Laptop_C_1_W=35, Laptop_C_1_On_a=64560, Laptop_C_1_Off_a=71100, Laptop_C_2_W=65, Laptop_C_2_On_a=0, Laptop_C_2_Off_a=23400, Laptop_C_2_On_b=79140, Laptop_C_2_Off_b=86400, Lamp_3_W=0, L3_On_1=0, L3_Off_1=0, L3_On_2=0, L3_Off_2=0, K_L6_W=40, K_L6_On_1=61800, K_L6_Off_1=70680, Heater_W=2000, Heater_On_1=60000, Heater_Off_1=70200, Stereo_W=40, Stereo_On_1=70320, Stereo_Off_1=77520, TV_W=300, TV_On_1=64440, TV_Off_1=70200, TV_On_2=0, TV_Off_2=0}
      mask prompts: V "Voltage"; t "Time"; K_L1_W "Light_1_W"; K_L1_On_1 "Light_1_On_a"; K_L1_Off_1 "Light_1_Off_a"; K_L1_On_2 "Light_1_On_b"; K_L1_Off_2 "Light_1_Off_b"; T_Initial "Fridge_Initial_Temp"; T_Low "Fridge_T_Low"; T_High "Fridge_T_High"; N_Elements "Oven_#_Elements"; Shower_L_a "Shower_Length_a"; Shower_L_b "Shower_Length_b"; Shower_L_c "Shower_Length_c"; K_L3_W "Light_3_W"; K_L3_On_1 "Light_3_On_a"; K_L3_Off_1 "Light_3_Off_a"; K_L3_On_2 "Light_3_On_b"; K_L3_Off_2 "Light_3_Off_b"; M "Mass of water"; D "HW_Diameter"; L "HW_Length"; Ti "Inlet Temp"; Ta "Ambient Temp"; L1_On_1 "Lamp_1_On_1"; L1_Off_1 "Lamp_1_Off_1"; L1_On_2 "Lamp_1_On_2"; L1_Off_2 "Lamp_1_Off_2"; K_L4_W "Light_4_W"; K_L4_On_1 "Light_4_On_a"; K_L4_Off_1 "Light_4_Off_a"; L2_On_1 "Lamp_2_On_1"; L2_Off_1 "Lamp_2_Off_1"; L2_On_2 "Lamp_2_On_2"; L2_Off_2 "Lamp_2_Off_2"; K_L5_W "Light_5_W"; K_L5_On_1 "Light_5_On_a"; K_L5_Off_1 "Light_5_Off_a"; K_L5_On_2 "Light_5_On_b"; K_L5_Off_2 "Light_5_Off_b"; L3_On_1 "Lamp_3_On_1"; L3_Off_1 "Lamp_3_Off_1"; L3_On_2 "Lamp_3_On_2"; L3_Off_2 "Lamp_3_Off_2"; K_L6_W "Light_6_W"; K_L6_On_1 "Light_6_On_a"; K_L6_Off_1 "Light_6_Off_a"
      mask DialogControl Container4 Prompt: House 1
      mask DialogControl Container10 Prompt: Kitchen
      mask DialogControl Container22 Prompt: Bathroom
      mask DialogControl Container20 Prompt: Bedroom 1
      mask DialogControl Container16 Prompt: Bedroom 2
      mask DialogControl Container11 Prompt: Lounge
      mask Display: image('house.jpg');
  Integrator : Integrator
  Line : Subsystem  mask{l=220}
      mask prompts: l "Length"
      mask Display: image('wire.png')
  PV : Subsystem  mask{P=6}
      mask prompts: P "Capacity (kW)"
      mask Display:
      | image('PV.jpg');
      | 
      | color('yellow')
      | port_label('input',1,'Solar Irr')
      | port_label('input',2,'BMS Connect')
      | port_label('output',2,'Output Power')
      | port_label('output',1,'Available Power')
  Product : Product
  Voltage Measurement : powerlib/Measurements/Voltage Measurement  PhasorSimulation=off  OutputType=Complex  PSBequivalent=0
  Wind Turbine : Subsystem  mask{V=240, W=1000}
      mask prompts: V "Voltage"; W "Watts"
      mask Display:
      | image('Wind.jpg')
      | 
      | color('green')
      | port_label('input',1,'Wind Speed')
      | port_label('input',2,'BMS Connect')
      | port_label('output',2,'Output Power')
      | port_label('output',1,'Available Power')
  powergui : powerlib/powergui  Priority=1  SimulationMode=Continuous  SampleTime=50e-6  frequency=60  Iterations=50  frequencyindice=60  Pbase=100e6  ErrMax=1e-4  UnitsV=kV  UnitsW=MW  buscounter=0  FunctionMessages=off  echomessages=off  EnableUseOfTLC=off  CurrentSourceSwitches=off  DisableSnubberDevices=off  DisableRonSwitches=off  DisableVfSwitches=off  DisplayEquations=off  AutomaticDiscreteSolvers=off  SolverType=Tustin/Backward Euler (TBE)  Interpol=off  ExternalGateDelay=off  methode=off  Ts=100  x0status=blocks  SPID=on  SwTol=0  HookPort=off  NonlinearTolerance=1e-4  nMaxIteration=100  ContinueOnMaxIteration=off  RestoreLinks=warning  ResistiveCurrentMeasurement=off  Frange=[0:2:500]  Ylog=off  Xlog=on  ShowGrid=off  save=off  variable=ZData  structure=0  ZoomFFT=off  StartTime=0.0  cycles=1  DisplayStyle=1  fundamental=60  FreqAxis=off  MaxFrequency=1000  frequencyindicesteady=1  RmsSteady=1  display=off
